simulink model "Cortina_RETO_FINAL"  (R2023b)
settings: Solver=VariableStepAuto  StartTime=0.0  StopTime=inf  RelTol=1e-3
settings Model: Creator=xhunter70  LastModifiedBy=Israe
settings SolverCC: ZeroCrossAlgorithm=Adaptive  AlgebraicLoopSolver=TrustRegion
settings DebuggingCC: IgnoredZcDiagnostic=none  UnconnectedInputMsg=warning  UnconnectedOutputMsg=warning  UnconnectedLineMsg=warning
settings ModelReferenceCC: EnableRefExpFcnMdlSchedulingChecks=on
settings RTWCC: DLArmComputeVersion=19.05

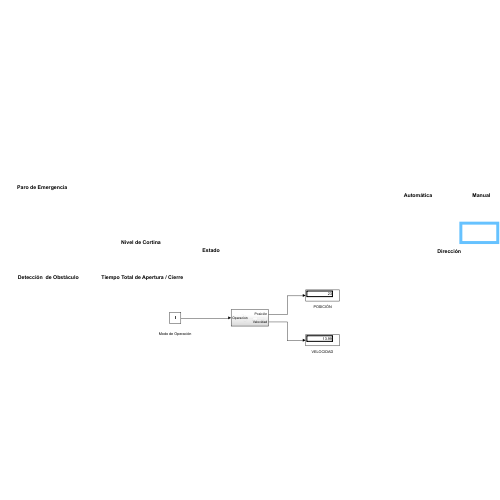
[thumbnail: Simulink's stored preview of the top-level diagram]
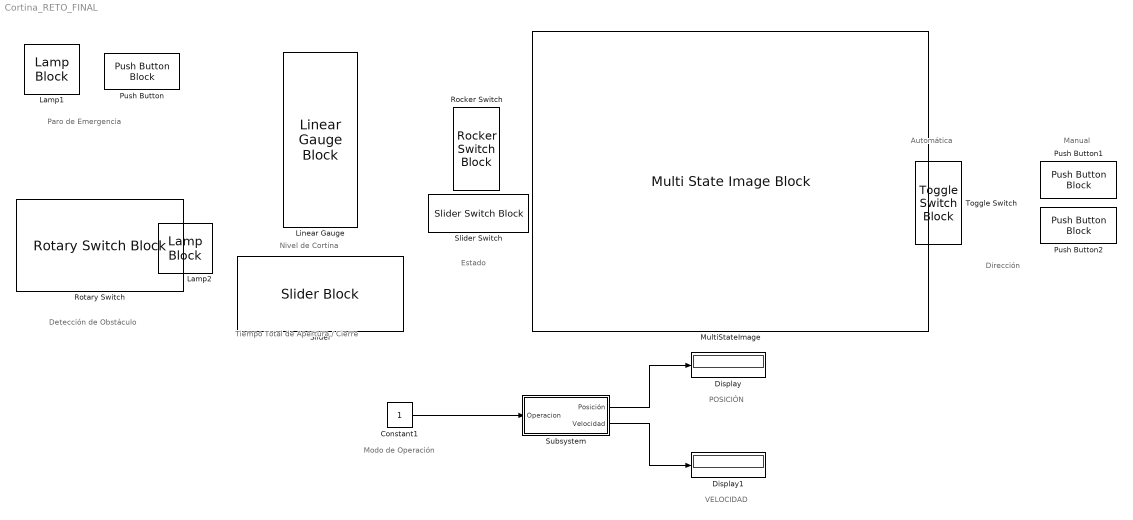
== system Cortina_RETO_FINAL ==
  note: VELOCIDAD
  note: POSICIÓN
  note: Modo de Operación
  note: Paro de Emergencia
  note: Dirección
  note: Estado
  note: Nivel de Cortina
  note: Tiempo Total de Apertura / Cierre
  note: Detección de Obstáculo
  note: Automática
  note: Manual
  Constant1 : Constant
  Display : Display
  Display1 : Display
  Lamp1 : LampBlock  LabelPosition=Hide  Icon=Hexagon
  Lamp2 : LampBlock  LabelPosition=Hide
  Linear Gauge : LinearGaugeBlock  ScaleMax=35
  MultiStateImage : MultiStateImageBlock  ScaleMode=Fixed
  Push Button : PushButtonBlock  ButtonText=STOP  OnValue=0
  Push Button1 : PushButtonBlock  ButtonText=UP
  Push Button2 : PushButtonBlock  ButtonText=DOWN  OnValue=-1  OffValue=0.000000
  Rocker Switch : RockerSwitchBlock  LabelPosition=Hide
      settings: Values={{'Automático', 'Manual'}, [0 1]}
  Rotary Switch : RotarySwitchBlock  LabelPosition=Hide
      settings: Values={{'Libre', 'Obstáculo Detectado'}, [0 1]}
  Slider : SliderBlock  LabelPosition=Hide  ScaleMin=3  ScaleMax=5
  Slider Switch : SliderSwitchBlock  LabelPosition=Hide
      settings: Values={{'Off', 'On'}, [0 1]}
  Subsystem : Subsystem
  Toggle Switch : ToggleSwitchBlock  LabelPosition=Hide
      settings: Values={{'Lower', 'Raise'}, [-1 1]}
  wires:
  Constant1/1 -> Subsystem/1(Operacion)
  Subsystem/1(Posición) -> Display/1
  Subsystem/2(Velocidad) -> Display1/1

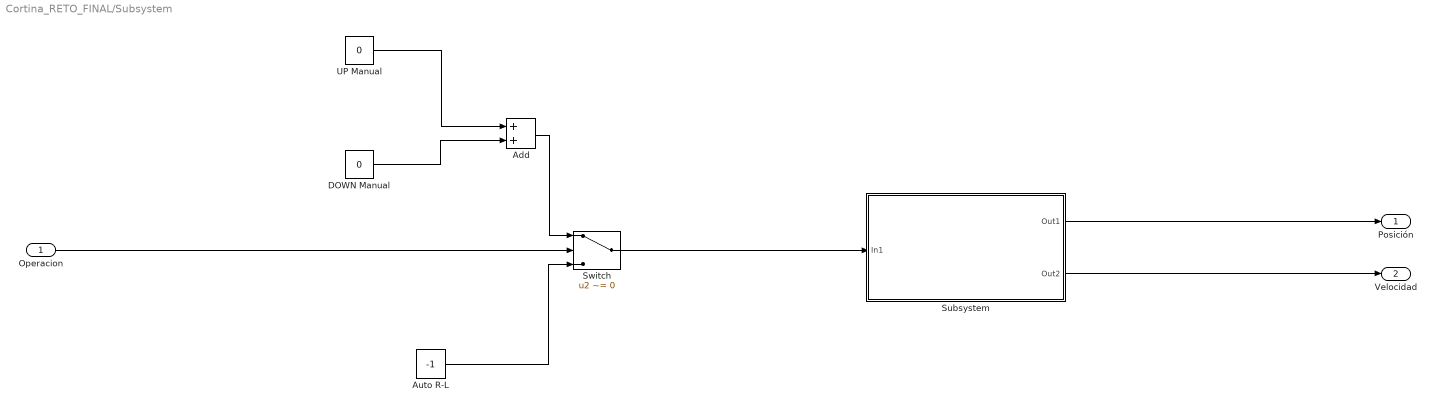
== system Cortina_RETO_FINAL/Subsystem ==
  Operacion : Inport
  Add : Sum
  Auto R-L : Constant  Value=-1
  DOWN Manual : Constant  Value=0
  Subsystem : Subsystem
  Switch : Switch  Criteria=u2 ~= 0  SaturateOnIntegerOverflow=off  InputSameDT=off
  UP Manual : Constant  Value=0
  Posición : Outport
  Velocidad : Outport  Port=2
  wires:
  Subsystem/1(Out1) -> Posición/1
  Subsystem/2(Out2) -> Velocidad/1
  Operacion/1 -> Switch/2
  Switch/1 -> Subsystem/1(In1)
  Auto R-L/1 -> Switch/3
  Add/1 -> Switch/1
  UP Manual/1 -> Add/1
  DOWN Manual/1 -> Add/2

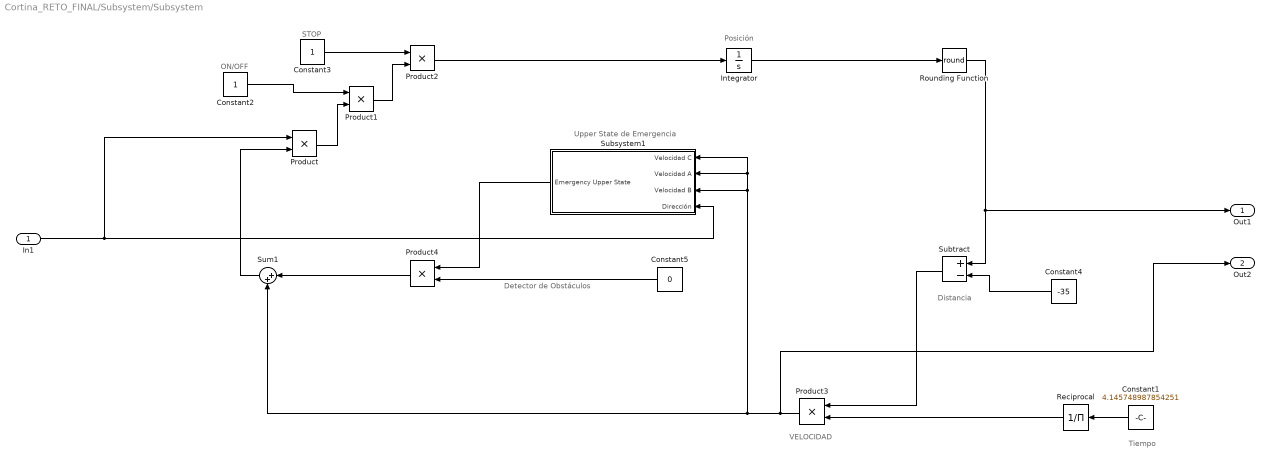
== system Cortina_RETO_FINAL/Subsystem/Subsystem ==
  note: STOP
  note: ON/OFF
  note: Tiempo
  note: Upper State de Emergencia
  note: Posición
  note: Detector de Obstáculos
  note: Distancia
  note: VELOCIDAD
  In1 : Inport
  Constant1 : Constant  Value=4.145748987854251
  Constant2 : Constant
  Constant3 : Constant
  Constant4 : Constant  Value=-35
  Constant5 : Constant  Value=0
  Integrator : Integrator  LimitOutput=on  UpperSaturationLimit=35  LowerSaturationLimit=0
  Product{,1..4} (5 alike) : Product
  Reciprocal : Product  Inputs=/
  Rounding Function : Rounding  Operator=round
  Subsystem1 : Subsystem
  Subtract : Sum  Inputs=+-
  Sum1 : Sum  Inputs=|++
  Out1 : Outport
  Out2 : Outport  Port=2
  wires:
  Constant3/1 -> Product2/1
  Sum1/1 -> Product/2
  Subtract/1 -> Product3/1
  Integrator/1 -> Rounding Function/1
  Constant1/1 -> Reciprocal/1
  In1/1 -> Subsystem1/4(Dirección), Product/1
  Product3/1 -> Out2/1, Subsystem1/1(Velocidad C), Subsystem1/2(Velocidad A), Subsystem1/3(Velocidad B), Sum1/2
  Product/1 -> Product1/2
  Product1/1 -> Product2/2
  Subsystem1/1(Emergency Upper State) -> Product4/1
  Reciprocal/1 -> Product3/2
  Constant2/1 -> Product1/1
  Rounding Function/1 -> Out1/1, Subtract/1
  Constant4/1 -> Subtract/2
  Constant5/1 -> Product4/2
  Product2/1 -> Integrator/1
  Product4/1 -> Sum1/1

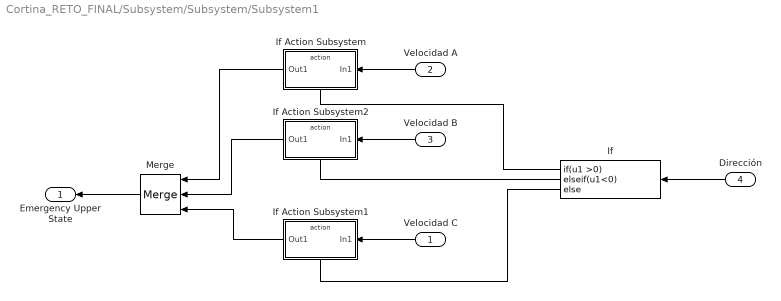
== system Cortina_RETO_FINAL/Subsystem/Subsystem/Subsystem1 ==
  Velocidad C : Inport
  Velocidad A : Inport  Port=2
  Velocidad B : Inport  Port=3
  Dirección : Inport  Port=4
  If : If  IfExpression=u1 >0  ElseIfExpressions=u1<0
  If Action Subsystem : Subsystem  TreatAsAtomicUnit=on
  If Action Subsystem1 : Subsystem  TreatAsAtomicUnit=on
  If Action Subsystem2 : Subsystem  TreatAsAtomicUnit=on
  Merge : Merge  Inputs=3
  Emergency Upper State : Outport
  wires:
  If Action Subsystem/1(Out1) -> Merge/1
  If/3 -> If Action Subsystem1/Action
  If Action Subsystem2/1(Out1) -> Merge/2
  If/2 -> If Action Subsystem2/Action
  If/1 -> If Action Subsystem/Action
  Merge/1 -> Emergency Upper State/1
  If Action Subsystem1/1(Out1) -> Merge/3
  Velocidad A/1 -> If Action Subsystem/1(In1)
  Velocidad B/1 -> If Action Subsystem2/1(In1)
  Velocidad C/1 -> If Action Subsystem1/1(In1)
  Dirección/1 -> If/1

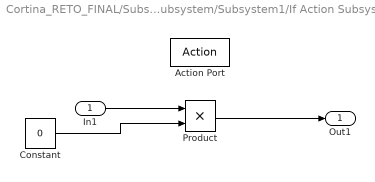
== system Cortina_RETO_FINAL/Subsystem/Subsystem/Subsystem1/If Action Subsystem ==
  In1 : Inport
  Action Port : ActionPort  ActionPortLabel=if(u1 >0)
  Constant : Constant  Value=0
  Product : Product
  Out1 : Outport
  wires:
  Product/1 -> Out1/1
  In1/1 -> Product/1
  Constant/1 -> Product/2

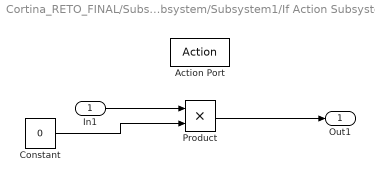
== system Cortina_RETO_FINAL/Subsystem/Subsystem/Subsystem1/If Action Subsystem1 ==
  In1 : Inport
  Action Port : ActionPort  ActionPortLabel=else
  Constant : Constant  Value=0
  Product : Product
  Out1 : Outport
  wires:
  In1/1 -> Product/1
  Product/1 -> Out1/1
  Constant/1 -> Product/2

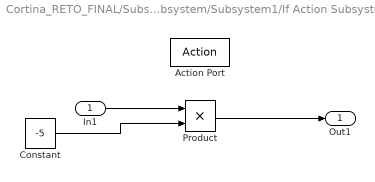
== system Cortina_RETO_FINAL/Subsystem/Subsystem/Subsystem1/If Action Subsystem2 ==
  In1 : Inport
  Action Port : ActionPort  ActionPortLabel=elseif(u1<0)
  Constant : Constant  Value=-5
  Product : Product
  Out1 : Outport
  wires:
  Product/1 -> Out1/1
  In1/1 -> Product/1
  Constant/1 -> Product/2
